simulink model "MX_Gateway"  (Simulink 9.0)
settings: Solver=FixedStepDiscrete  StartTime=0.0  StopTime=100  FixedStep=0.01  RelTol=1e-3
values from neighbouring files (symbols this model uses):
  ACTIVE = Bobcat.Parameter; CreateDefine( ACTIVE                         ,1                          ,'Define' ,'defines.h' )    [defines.m]
  ACTIVE_MODE_ONE = Bobcat.Parameter; CreateDefine( ACTIVE_MODE_ONE                ,19                         ,'Define' ,'defines.h' )    [defines.m]
  AUTHENTICATED = Bobcat.Parameter; CreateDefine( AUTHENTICATED                  ,1                          ,'Define' ,'defines.h' )    [defines.m]
  AUX_EMEA_CONTROLS = Bobcat.Parameter; CreateDefine( AUX_EMEA_CONTROLS  ,1   ,'Define' ,'defines.h' )    [enums.m]
  AUX_FLOW_HIGH = Bobcat.Parameter; CreateDefine( AUX_FLOW_HIGH           ,4  ,'Define' ,'defines.h' )    [enums.m]
  AUX_FLOW_INACTIVE = Bobcat.Parameter; CreateDefine( AUX_FLOW_INACTIVE       ,0  ,'Define' ,'defines.h' )    [enums.m]
  AUX_FLOW_LOW = Bobcat.Parameter; CreateDefine( AUX_FLOW_LOW            ,1  ,'Define' ,'defines.h' )    [enums.m]
  AUX_FLOW_MEDIUM = Bobcat.Parameter; CreateDefine( AUX_FLOW_MEDIUM         ,2  ,'Define' ,'defines.h' )    [enums.m]
  AUX_FLOW_STANDARD = Bobcat.Parameter; CreateDefine( AUX_FLOW_STANDARD       ,3  ,'Define' ,'defines.h' )    [enums.m]
  AUX_NON_FUNCTIONAL = Bobcat.Parameter; CreateDefine( AUX_NON_FUNCTIONAL ,255 ,'Define' ,'defines.h' )    [enums.m]
  BASE_ACTIVE = Bobcat.Parameter; CreateDefine( BASE_ACTIVE                    ,253                        ,'Define' ,'defines.h' )    [defines.m]
  BASE_DETENT = Bobcat.Parameter; CreateDefine( BASE_DETENT                    ,1                          ,'Define' ,'defines.h' )    [defines.m]
  BEEP_NONE = Bobcat.Parameter; CreateDefine( BEEP_NONE                      ,255                        ,'Define' ,'defines.h' )    [defines.m]
  CD_AIR_FILTER_DEBOUNCE_NORMAL = Bobcat.Parameter; CreateAliasCD( CD_AIR_FILTER_DEBOUNCE_NORMAL                        ,uint16(500)                ,'ConstantDataPointer' ,'CONSTANT_DATA' ,'AIR_FILTER_DEBOUNCE_NORMAL')    [cdata.m]    (also in hc12_cdata.m; m4_cdata.m)
  CD_AIR_FILTER_DEBOUNCE_NOT_CONNECTED = Bobcat.Parameter; CreateAliasCD( CD_AIR_FILTER_DEBOUNCE_NOT_CONNECTED                 ,uint16(3000)               ,'ConstantDataPointer' ,'CONSTANT_DATA' ,'AIR_FILTER_DEBOUNCE_NOT_CONNECTED')    [cdata.m]    (also in hc12_cdata.m; m4_cdata.m)
  CD_AIR_FILTER_DEBOUNCE_PLUGGED = Bobcat.Parameter; CreateAliasCD( CD_AIR_FILTER_DEBOUNCE_PLUGGED                       ,uint16(3000)               ,'ConstantDataPointer' ,'CONSTANT_DATA' ,'AIR_FILTER_DEBOUNCE_PLUGGED')    [cdata.m]    (also in hc12_cdata.m; m4_cdata.m)
  CD_AIR_FILTER_FUNCTION = Bobcat.Parameter; CreateAliasCD( CD_AIR_FILTER_FUNCTION                               ,uint8(255)                 ,'ConstantDataPointer' ,'CONSTANT_DATA' ,'AIR_FILTER_FUNCTION')    [cdata.m]    (also in hc12_cdata.m; m4_cdata.m)
  CD_AIR_FILTER_PLUGGED_WEIGHT = Bobcat.Parameter; CreateAliasCD( CD_AIR_FILTER_PLUGGED_WEIGHT                         ,uint8(2)                   ,'ConstantDataPointer' ,'CONSTANT_DATA' ,'AIR_FILTER_PLUGGED_WEIGHT')    [cdata.m]    (also in hc12_cdata.m; m4_cdata.m)
  CD_AIR_FILTER_UNPLUGGED_WEIGHT = Bobcat.Parameter; CreateAliasCD( CD_AIR_FILTER_UNPLUGGED_WEIGHT                       ,uint8(1)                   ,'ConstantDataPointer' ,'CONSTANT_DATA' ,'AIR_FILTER_UNPLUGGED_WEIGHT')    [cdata.m]    (also in hc12_cdata.m; m4_cdata.m)
  CD_AUTOIDLE_ARMBAR_DELAY = Bobcat.Parameter; CreateAliasCD( CD_AUTOIDLE_ARMBAR_DELAY                             ,uint8(2)                   ,'ConstantDataPointer' ,'CONSTANT_DATA' ,'AUTOIDLE_ARMBAR_DELAY')    [cdata.m]    (also in hc12_cdata.m; m4_cdata.m)
  CD_AUTOIDLE_FUNCTION = Bobcat.Parameter; CreateAliasCD( CD_AUTOIDLE_FUNCTION                                 ,uint8(255)                 ,'ConstantDataPointer' ,'CONSTANT_DATA' ,'AUTOIDLE_FUNCTION')    [cdata.m]    (also in hc12_cdata.m; m4_cdata.m)
  CD_AUTOIDLE_IDLE_DELAY = Bobcat.Parameter; CreateAliasCD( CD_AUTOIDLE_IDLE_DELAY                               ,uint8(40)                  ,'ConstantDataPointer' ,'CONSTANT_DATA' ,'AUTOIDLE_IDLE_DELAY')    [cdata.m]    (also in hc12_cdata.m; m4_cdata.m)
  CD_AUTOIDLE_LOW_IDLE_START = Bobcat.Parameter; CreateAliasCD( CD_AUTOIDLE_LOW_IDLE_START                           ,uint8(255)                 ,'ConstantDataPointer' ,'CONSTANT_DATA' ,'AUTOIDLE_LOW_IDLE_START')    [cdata.m]    (also in hc12_cdata.m; m4_cdata.m)
  CD_AUTOIDLE_PRESSURE_OFF = Bobcat.Parameter; CreateAliasCD( CD_AUTOIDLE_PRESSURE_OFF                             ,uint16(300)                ,'ConstantDataPointer' ,'CONSTANT_DATA' ,'AUTOIDLE_PRESSURE_OFF')    [cdata.m]    (also in hc12_cdata.m; m4_cdata.m)
  CD_AUTOIDLE_PRESSURE_ON = Bobcat.Parameter; CreateAliasCD( CD_AUTOIDLE_PRESSURE_ON                              ,uint16(270)                ,'ConstantDataPointer' ,'CONSTANT_DATA' ,'AUTOIDLE_PRESSURE_ON')    [cdata.m]    (also in hc12_cdata.m; m4_cdata.m)
  CD_AUTOSHIFT_FUNCTION = Bobcat.Parameter; CreateAliasCD( CD_AUTOSHIFT_FUNCTION                                ,uint8(255)                 ,'ConstantDataPointer' ,'CONSTANT_DATA' ,'AUTOSHIFT_FUNCTION')    [cdata.m]    (also in hc12_cdata.m; m4_cdata.m)
  CD_AUTOSHIFT_PHIGH1 = Bobcat.Parameter; CreateAliasCD( CD_AUTOSHIFT_PHIGH1                                  ,uint16(205)                ,'ConstantDataPointer' ,'CONSTANT_DATA' ,'AUTOSHIFT_PHIGH1')    [cdata.m]    (also in hc12_cdata.m; m4_cdata.m)
  CD_AUTOSHIFT_PHIGH2 = Bobcat.Parameter; CreateAliasCD( CD_AUTOSHIFT_PHIGH2                                  ,uint16(193)                ,'ConstantDataPointer' ,'CONSTANT_DATA' ,'AUTOSHIFT_PHIGH2')    [cdata.m]    (also in hc12_cdata.m; m4_cdata.m)
  CD_AUTOSHIFT_PHIGH_TEMP1 = Bobcat.Parameter; CreateAliasCD( CD_AUTOSHIFT_PHIGH_TEMP1                             ,int16(70)                  ,'ConstantDataPointer' ,'CONSTANT_DATA' ,'AUTOSHIFT_PHIGH_TEMP1')    [cdata.m]    (also in hc12_cdata.m; m4_cdata.m)
  CD_AUTOSHIFT_PHIGH_TEMP2 = Bobcat.Parameter; CreateAliasCD( CD_AUTOSHIFT_PHIGH_TEMP2                             ,int16(160)                 ,'ConstantDataPointer' ,'CONSTANT_DATA' ,'AUTOSHIFT_PHIGH_TEMP2')    [cdata.m]    (also in hc12_cdata.m; m4_cdata.m)
  CD_AUTOSHIFT_PLOW1 = Bobcat.Parameter; CreateAliasCD( CD_AUTOSHIFT_PLOW1                                   ,uint16(130)                ,'ConstantDataPointer' ,'CONSTANT_DATA' ,'AUTOSHIFT_PLOW1')    [cdata.m]    (also in hc12_cdata.m; m4_cdata.m)
  CD_AUTOSHIFT_PLOW2 = Bobcat.Parameter; CreateAliasCD( CD_AUTOSHIFT_PLOW2                                   ,uint16(100)                ,'ConstantDataPointer' ,'CONSTANT_DATA' ,'AUTOSHIFT_PLOW2')    [cdata.m]    (also in hc12_cdata.m; m4_cdata.m)
  CD_AUTOSHIFT_PLOW_TEMP1 = Bobcat.Parameter; CreateAliasCD( CD_AUTOSHIFT_PLOW_TEMP1                              ,int16(20)                  ,'ConstantDataPointer' ,'CONSTANT_DATA' ,'AUTOSHIFT_PLOW_TEMP1')    [cdata.m]    (also in hc12_cdata.m; m4_cdata.m)
  CD_AUTOSHIFT_PLOW_TEMP2 = Bobcat.Parameter; CreateAliasCD( CD_AUTOSHIFT_PLOW_TEMP2                              ,int16(80)                  ,'ConstantDataPointer' ,'CONSTANT_DATA' ,'AUTOSHIFT_PLOW_TEMP2')    [cdata.m]    (also in hc12_cdata.m; m4_cdata.m)
  CD_AUTOSHIFT_TRAVEL_DELAY = Bobcat.Parameter; CreateAliasCD( CD_AUTOSHIFT_TRAVEL_DELAY                            ,uint8(32)                  ,'ConstantDataPointer' ,'CONSTANT_DATA' ,'AUTOSHIFT_TRAVEL_DELAY')    [cdata.m]    (also in hc12_cdata.m; m4_cdata.m)
  CD_AUX2_CRACKPOINT_CURRENT = Bobcat.Parameter; CreateAliasCD( CD_AUX2_CRACKPOINT_CURRENT                           ,fi(0,0,16,12,'hex','0c28') ,'ConstantDataPointer' ,'CONSTANT_DATA' ,'AUX2_CRACKPOINT_CURRENT')    [cdata.m]    (also in hc12_cdata.m; m4_cdata.m)
  CD_AUX2_FLOW_LOW_FUNCTION = Bobcat.Parameter; CreateAliasCD( CD_AUX2_FLOW_LOW_FUNCTION                            ,uint8(255)                 ,'ConstantDataPointer' ,'CONSTANT_DATA' ,'AUX2_FLOW_LOW_FUNCTION')    [cdata.m]    (also in hc12_cdata.m; m4_cdata.m)
  CD_AUX2_FLOW_MED_FUNCTION = Bobcat.Parameter; CreateAliasCD( CD_AUX2_FLOW_MED_FUNCTION                            ,uint8(255)                 ,'ConstantDataPointer' ,'CONSTANT_DATA' ,'AUX2_FLOW_MED_FUNCTION')    [cdata.m]    (also in hc12_cdata.m; m4_cdata.m)
  CD_AUX2_HI_FLOW_PERCENT = Bobcat.Parameter; CreateAliasCD( CD_AUX2_HI_FLOW_PERCENT                              ,fi(0,0,16,16,'hex','ffff') ,'ConstantDataPointer' ,'CONSTANT_DATA' ,'AUX2_HI_FLOW_PERCENT')    [cdata.m]    (also in hc12_cdata.m; m4_cdata.m)
  CD_AUX2_LOW_FLOW_PERCENT = Bobcat.Parameter; CreateAliasCD( CD_AUX2_LOW_FLOW_PERCENT                             ,fi(0,0,16,16,'hex','7333') ,'ConstantDataPointer' ,'CONSTANT_DATA' ,'AUX2_LOW_FLOW_PERCENT')    [cdata.m]    (also in hc12_cdata.m; m4_cdata.m)
  CD_AUX2_MAX_FLOW_CURRENT = Bobcat.Parameter; CreateAliasCD( CD_AUX2_MAX_FLOW_CURRENT                             ,fi(0,0,16,12,'hex','14cc') ,'ConstantDataPointer' ,'CONSTANT_DATA' ,'AUX2_MAX_FLOW_CURRENT')    [cdata.m]    (also in hc12_cdata.m; m4_cdata.m)
  CD_AUX2_MED_FLOW_PERCENT = Bobcat.Parameter; CreateAliasCD( CD_AUX2_MED_FLOW_PERCENT                             ,fi(0,0,16,16,'hex','9999') ,'ConstantDataPointer' ,'CONSTANT_DATA' ,'AUX2_MED_FLOW_PERCENT')    [cdata.m]    (also in hc12_cdata.m; m4_cdata.m)
  CD_AUX_CRACKPOINT_CURRENT = Bobcat.Parameter; CreateAliasCD( CD_AUX_CRACKPOINT_CURRENT                            ,fi(0,0,16,12,'hex','0000') ,'ConstantDataPointer' ,'CONSTANT_DATA' ,'AUX_CRACKPOINT_CURRENT')    [cdata.m]    (also in hc12_cdata.m; m4_cdata.m)
  CD_AUX_DETENT_FLASH_TIME = Bobcat.Parameter; CreateAliasCD( CD_AUX_DETENT_FLASH_TIME                             ,uint16(72)                 ,'ConstantDataPointer' ,'CONSTANT_DATA' ,'AUX_DETENT_FLASH_TIME')    [cdata.m]    (also in hc12_cdata.m; m4_cdata.m)
  CD_AUX_DETENT_HOLD_TIME = Bobcat.Parameter; CreateAliasCD( CD_AUX_DETENT_HOLD_TIME                              ,uint16(100)                ,'ConstantDataPointer' ,'CONSTANT_DATA' ,'AUX_DETENT_HOLD_TIME')    [cdata.m]    (also in hc12_cdata.m; m4_cdata.m)
  CD_AUX_FULL_ON_AUX1 = Bobcat.Parameter; CreateAliasCD( CD_AUX_FULL_ON_AUX1                                  ,fi(0,0,16,12,'hex','10cd') ,'ConstantDataPointer' ,'CONSTANT_DATA' ,'AUX_FULL_ON_AUX1')    [cdata.m]    (also in hc12_cdata.m; m4_cdata.m)
  CD_AUX_FULL_ON_AUX2 = Bobcat.Parameter; CreateAliasCD( CD_AUX_FULL_ON_AUX2                                  ,fi(0,0,16,12,'hex','139d') ,'ConstantDataPointer' ,'CONSTANT_DATA' ,'AUX_FULL_ON_AUX2')    [cdata.m]    (also in hc12_cdata.m; m4_cdata.m)
  CD_AUX_FULL_ON_AUX3 = Bobcat.Parameter; CreateAliasCD( CD_AUX_FULL_ON_AUX3                                  ,fi(0,0,16,12,'hex','17d7') ,'ConstantDataPointer' ,'CONSTANT_DATA' ,'AUX_FULL_ON_AUX3')    [cdata.m]    (also in hc12_cdata.m; m4_cdata.m)
  CD_AUX_FUNCTION = Bobcat.Parameter; CreateAliasCD( CD_AUX_FUNCTION                                      ,uint8(0)                   ,'ConstantDataPointer' ,'CONSTANT_DATA' ,'AUX_FUNCTION')    [cdata.m]    (also in hc12_cdata.m; m4_cdata.m)
  CD_AUX_RATE_LIMIT_CRACKPOINT = Bobcat.Parameter; CreateAliasCD( CD_AUX_RATE_LIMIT_CRACKPOINT                         ,fi(0,0,16,12,'hex','0800') ,'ConstantDataPointer' ,'CONSTANT_DATA' ,'AUX_RATE_LIMIT_CRACKPOINT')    [cdata.m]    (also in hc12_cdata.m; m4_cdata.m)
  CD_AUX_RATE_LIMIT_CRACKPOINT_RATE = Bobcat.Parameter; CreateAliasCD( CD_AUX_RATE_LIMIT_CRACKPOINT_RATE                    ,fi(0,1,32,12,'int',102400) ,'ConstantDataPointer' ,'CONSTANT_DATA' ,'AUX_RATE_LIMIT_CRACKPOINT_RATE')    [cdata.m]    (also in hc12_cdata.m; m4_cdata.m)
  CD_AUX_RATE_LIMIT_FUNCTION = Bobcat.Parameter; CreateAliasCD( CD_AUX_RATE_LIMIT_FUNCTION                           ,uint8(255)                 ,'ConstantDataPointer' ,'CONSTANT_DATA' ,'AUX_RATE_LIMIT_FUNCTION')    [cdata.m]    (also in hc12_cdata.m; m4_cdata.m)
  CD_AUX_RATE_LIMIT_MAX_ENG_SPD = Bobcat.Parameter; CreateAliasCD( CD_AUX_RATE_LIMIT_MAX_ENG_SPD                        ,uint16(1600)               ,'ConstantDataPointer' ,'CONSTANT_DATA' ,'AUX_RATE_LIMIT_MAX_ENG_SPD')    [cdata.m]    (also in hc12_cdata.m; m4_cdata.m)
  CD_AUX_RATE_LIMIT_MIN_ENG_SPD = Bobcat.Parameter; CreateAliasCD( CD_AUX_RATE_LIMIT_MIN_ENG_SPD                        ,uint16(100)                ,'ConstantDataPointer' ,'CONSTANT_DATA' ,'AUX_RATE_LIMIT_MIN_ENG_SPD')    [cdata.m]    (also in hc12_cdata.m; m4_cdata.m)
  CD_AUX_RATE_LIMIT_NORMAL_RATE = Bobcat.Parameter; CreateAliasCD( CD_AUX_RATE_LIMIT_NORMAL_RATE                        ,fi(0,1,32,12,'int',2048)   ,'ConstantDataPointer' ,'CONSTANT_DATA' ,'AUX_RATE_LIMIT_NORMAL_RATE')    [cdata.m]    (also in hc12_cdata.m; m4_cdata.m)
  CD_AUXPOT_CAL_DEADBAND_HIGH = Bobcat.Parameter; CreateAliasCD( CD_AUXPOT_CAL_DEADBAND_HIGH                          ,uint16(574)                ,'ConstantDataPointer' ,'CONSTANT_DATA' ,'AUXPOT_CAL_DEADBAND_HIGH')    [cdata.m]    (also in hc12_cdata.m; m4_cdata.m)
  CD_AUXPOT_CAL_DEADBAND_LOW = Bobcat.Parameter; CreateAliasCD( CD_AUXPOT_CAL_DEADBAND_LOW                           ,uint16(424)                ,'ConstantDataPointer' ,'CONSTANT_DATA' ,'AUXPOT_CAL_DEADBAND_LOW')    [cdata.m]    (also in hc12_cdata.m; m4_cdata.m)
  CD_AUXPOT_CENTER_RATIO = Bobcat.Parameter; CreateAliasCD( CD_AUXPOT_CENTER_RATIO                               ,uint16(500)                ,'ConstantDataPointer' ,'CONSTANT_DATA' ,'AUXPOT_CENTER_RATIO')    [cdata.m]    (also in hc12_cdata.m; m4_cdata.m)
  CD_AUXPOT_DEADBAND = Bobcat.Parameter; CreateAliasCD( CD_AUXPOT_DEADBAND                                   ,uint8(38)                  ,'ConstantDataPointer' ,'CONSTANT_DATA' ,'AUXPOT_DEADBAND')    [cdata.m]    (also in hc12_cdata.m; m4_cdata.m)
  CD_AUXPOT_FUNCTION = Bobcat.Parameter; CreateAliasCD( CD_AUXPOT_FUNCTION                                   ,uint8(0)                   ,'ConstantDataPointer' ,'CONSTANT_DATA' ,'AUXPOT_FUNCTION')    [cdata.m]    (also in hc12_cdata.m; m4_cdata.m)
  CD_AUXPOT_RATIO_GAIN = Bobcat.Parameter; CreateAliasCD( CD_AUXPOT_RATIO_GAIN                                 ,uint16(1000)               ,'ConstantDataPointer' ,'CONSTANT_DATA' ,'AUXPOT_RATIO_GAIN')    [cdata.m]    (also in hc12_cdata.m; m4_cdata.m)
  CD_CAN_THROTTLE_FUNCTION = Bobcat.Parameter; CreateAliasCD( CD_CAN_THROTTLE_FUNCTION                             ,uint8(255)                 ,'ConstantDataPointer' ,'CONSTANT_DATA' ,'CAN_THROTTLE_FUNCTION')    [cdata.m]    (also in hc12_cdata.m; m4_cdata.m)
  CD_CAN_THROTTLE_REVERSE = Bobcat.Parameter; CreateAliasCD( CD_CAN_THROTTLE_REVERSE                              ,uint8(255)                 ,'ConstantDataPointer' ,'CONSTANT_DATA' ,'CAN_THROTTLE_REVERSE')    [cdata.m]    (also in hc12_cdata.m; m4_cdata.m)
  CD_CONSOLE_DEBOUNCE_ABSENT = Bobcat.Parameter; CreateAliasCD( CD_CONSOLE_DEBOUNCE_ABSENT                           ,uint16(12)                 ,'ConstantDataPointer' ,'CONSTANT_DATA' ,'CONSOLE_DEBOUNCE_ABSENT')    [cdata.m]    (also in hc12_cdata.m; m4_cdata.m)
  CD_CONSOLE_DEBOUNCE_OORH = Bobcat.Parameter; CreateAliasCD( CD_CONSOLE_DEBOUNCE_OORH                             ,uint16(12)                 ,'ConstantDataPointer' ,'CONSTANT_DATA' ,'CONSOLE_DEBOUNCE_OORH')    [cdata.m]    (also in hc12_cdata.m; m4_cdata.m)
  CD_CONSOLE_DEBOUNCE_OORL = Bobcat.Parameter; CreateAliasCD( CD_CONSOLE_DEBOUNCE_OORL                             ,uint16(12)                 ,'ConstantDataPointer' ,'CONSTANT_DATA' ,'CONSOLE_DEBOUNCE_OORL')    [cdata.m]    (also in hc12_cdata.m; m4_cdata.m)
  CD_CONSOLE_DEBOUNCE_PRESENT = Bobcat.Parameter; CreateAliasCD( CD_CONSOLE_DEBOUNCE_PRESENT                          ,uint16(12)                 ,'ConstantDataPointer' ,'CONSTANT_DATA' ,'CONSOLE_DEBOUNCE_PRESENT')    [cdata.m]    (also in hc12_cdata.m; m4_cdata.m)
  CD_CONSOLE_FUNCTION = Bobcat.Parameter; CreateAliasCD( CD_CONSOLE_FUNCTION                                  ,uint8(0)                   ,'ConstantDataPointer' ,'CONSTANT_DATA' ,'CONSOLE_FUNCTION')    [cdata.m]    (also in hc12_cdata.m; m4_cdata.m)
  CD_CONSOLE_OORH = Bobcat.Parameter; CreateAliasCD( CD_CONSOLE_OORH                                      ,uint16(740)                ,'ConstantDataPointer' ,'CONSTANT_DATA' ,'CONSOLE_OORH')    [cdata.m]    (also in hc12_cdata.m; m4_cdata.m)
  CD_CONSOLE_OORL = Bobcat.Parameter; CreateAliasCD( CD_CONSOLE_OORL                                      ,uint16(10)                 ,'ConstantDataPointer' ,'CONSTANT_DATA' ,'CONSOLE_OORL')    [cdata.m]    (also in hc12_cdata.m; m4_cdata.m)
  CD_CONSOLE_PRESENT_HIGH = Bobcat.Parameter; CreateAliasCD( CD_CONSOLE_PRESENT_HIGH                              ,uint16(515)                ,'ConstantDataPointer' ,'CONSTANT_DATA' ,'CONSOLE_PRESENT_HIGH')    [cdata.m]    (also in hc12_cdata.m; m4_cdata.m)
  CD_CONSOLE_PRESENT_LOW = Bobcat.Parameter; CreateAliasCD( CD_CONSOLE_PRESENT_LOW                               ,uint16(375)                ,'ConstantDataPointer' ,'CONSTANT_DATA' ,'CONSOLE_PRESENT_LOW')    [cdata.m]    (also in hc12_cdata.m; m4_cdata.m)
  CD_DELUXE_GEN5_5_FUNCTION = Bobcat.Parameter; CreateAliasCD( CD_DELUXE_GEN5_5_FUNCTION                            ,uint8(255)                 ,'ConstantDataPointer' ,'CONSTANT_DATA' ,'DELUXE_GEN5_5_FUNCTION')    [cdata.m]    (also in hc12_cdata.m; m4_cdata.m)
  CD_DELUXE_GEN5_FUNCTION = Bobcat.Parameter; CreateAliasCD( CD_DELUXE_GEN5_FUNCTION                              ,uint8(255)                 ,'ConstantDataPointer' ,'CONSTANT_DATA' ,'DELUXE_GEN5_FUNCTION')    [cdata.m]    (also in hc12_cdata.m; m4_cdata.m)
  CD_DERATE_ENGINE_SPEED_DEBOUNCE_HIGH = Bobcat.Parameter; CreateAliasCD( CD_DERATE_ENGINE_SPEED_DEBOUNCE_HIGH                 ,uint16(200)                ,'ConstantDataPointer' ,'CONSTANT_DATA' ,'DERATE_ENGINE_SPEED_DEBOUNCE_HIGH')    [cdata.m]    (also in hc12_cdata.m; m4_cdata.m)
  CD_DERATE_ENGINE_SPEED_DEBOUNCE_SHUTDOWN = Bobcat.Parameter; CreateAliasCD( CD_DERATE_ENGINE_SPEED_DEBOUNCE_SHUTDOWN             ,uint16(1000)               ,'ConstantDataPointer' ,'CONSTANT_DATA' ,'DERATE_ENGINE_SPEED_DEBOUNCE_SHUTDOWN')    [cdata.m]    (also in hc12_cdata.m; m4_cdata.m)
  CD_DERATE_ENGINE_SPEED_HIGH_OFFSET = Bobcat.Parameter; CreateAliasCD( CD_DERATE_ENGINE_SPEED_HIGH_OFFSET                   ,uint16(200)                ,'ConstantDataPointer' ,'CONSTANT_DATA' ,'DERATE_ENGINE_SPEED_HIGH_OFFSET')    [cdata.m]    (also in hc12_cdata.m; m4_cdata.m)
  CD_DERATE_METHOD = Bobcat.Parameter; CreateAliasCD( CD_DERATE_METHOD                                     ,uint8(0)                   ,'ConstantDataPointer' ,'CONSTANT_DATA' ,'DERATE_METHOD')    [cdata.m]    (also in hc12_cdata.m; m4_cdata.m)
  CD_DUMP_VALVE_FUNCTION = Bobcat.Parameter; CreateAliasCD( CD_DUMP_VALVE_FUNCTION                               ,uint8(255)                 ,'ConstantDataPointer' ,'CONSTANT_DATA' ,'DUMP_VALVE_FUNCTION')    [cdata.m]    (also in hc12_cdata.m; m4_cdata.m)
  CD_DUMP_VALVE_ON_CURRENT = Bobcat.Parameter; CreateAliasCD( CD_DUMP_VALVE_ON_CURRENT                             ,fi(0,0,16,12,'hex','ffff') ,'ConstantDataPointer' ,'CONSTANT_DATA' ,'DUMP_VALVE_ON_CURRENT')    [cdata.m]    (also in hc12_cdata.m; m4_cdata.m)
  CD_ECO_MODE_THROTTLE_REDUCTION = Bobcat.Parameter; CreateAliasCD( CD_ECO_MODE_THROTTLE_REDUCTION                       ,uint16(850)                ,'ConstantDataPointer' ,'CONSTANT_DATA' ,'ECO_MODE_THROTTLE_REDUCTION')    [cdata.m]    (also in hc12_cdata.m; m4_cdata.m)
  CD_ECT_FILTER_GAIN = Bobcat.Parameter; CreateAliasCD( CD_ECT_FILTER_GAIN                                   ,uint8(6)                   ,'ConstantDataPointer' ,'CONSTANT_DATA' ,'ECT_FILTER_GAIN')    [cdata.m]    (also in hc12_cdata.m; m4_cdata.m)
  CD_ECU_FUNCTION = Bobcat.Parameter; CreateAliasCD( CD_ECU_FUNCTION                                      ,uint8(255)                 ,'ConstantDataPointer' ,'CONSTANT_DATA' ,'ECU_FUNCTION')    [cdata.m]    (also in hc12_cdata.m; m4_cdata.m)
  CD_ECU_HOLD_DEBOUNCE_ERROR_ON = Bobcat.Parameter; CreateAliasCD( CD_ECU_HOLD_DEBOUNCE_ERROR_ON                        ,uint16(20)                 ,'ConstantDataPointer' ,'CONSTANT_DATA' ,'ECU_HOLD_DEBOUNCE_ERROR_ON')    [cdata.m]    (also in hc12_cdata.m; m4_cdata.m)
  CD_EMEA_CONTROLS_HOLD_TIME = Bobcat.Parameter; CreateAliasCD( CD_EMEA_CONTROLS_HOLD_TIME                           ,uint16(30)                 ,'ConstantDataPointer' ,'CONSTANT_DATA' ,'EMEA_CONTROLS_HOLD_TIME')    [cdata.m]    (also in hc12_cdata.m; m4_cdata.m)
  CD_EMEA_CONTROLS_OPTION_A = Bobcat.Parameter; CreateAliasCD( CD_EMEA_CONTROLS_OPTION_A                            ,uint8(255)                 ,'ConstantDataPointer' ,'CONSTANT_DATA' ,'EMEA_CONTROLS_OPTION_A')    [cdata.m]    (also in hc12_cdata.m; m4_cdata.m)
  CD_EMEA_CONTROLS_OPTION_C = Bobcat.Parameter; CreateAliasCD( CD_EMEA_CONTROLS_OPTION_C                            ,uint8(255)                 ,'ConstantDataPointer' ,'CONSTANT_DATA' ,'EMEA_CONTROLS_OPTION_C')    [cdata.m]    (also in hc12_cdata.m; m4_cdata.m)
  CD_EMEA_CONTROLS_REVERSE = Bobcat.Parameter; CreateAliasCD( CD_EMEA_CONTROLS_REVERSE                             ,uint8(255)                 ,'ConstantDataPointer' ,'CONSTANT_DATA' ,'EMEA_CONTROLS_REVERSE')    [cdata.m]    (also in hc12_cdata.m; m4_cdata.m)
  CD_EMEA_ELECTRIC_VERSION = Bobcat.Parameter; CreateAliasCD( CD_EMEA_ELECTRIC_VERSION                             ,uint8(0)                   ,'ConstantDataPointer' ,'CONSTANT_DATA' ,'EMEA_ELECTRIC_VERSION')    [cdata.m]    (also in hc12_cdata.m; m4_cdata.m)
  CD_EMEA_POT_CAL_DEADBAND_HIGH = Bobcat.Parameter; CreateAliasCD( CD_EMEA_POT_CAL_DEADBAND_HIGH                        ,uint16(574)                ,'ConstantDataPointer' ,'CONSTANT_DATA' ,'EMEA_POT_CAL_DEADBAND_HIGH')    [cdata.m]    (also in hc12_cdata.m; m4_cdata.m)
  CD_EMEA_POT_CAL_DEADBAND_LOW = Bobcat.Parameter; CreateAliasCD( CD_EMEA_POT_CAL_DEADBAND_LOW                         ,uint16(424)                ,'ConstantDataPointer' ,'CONSTANT_DATA' ,'EMEA_POT_CAL_DEADBAND_LOW')    [cdata.m]    (also in hc12_cdata.m; m4_cdata.m)
  CD_ENGINE_RPM_FACTOR = Bobcat.Parameter; CreateAliasCD( CD_ENGINE_RPM_FACTOR                                 ,uint8(35)                  ,'ConstantDataPointer' ,'CONSTANT_DATA' ,'ENGINE_RPM_FACTOR')    [cdata.m]    (also in hc12_cdata.m; m4_cdata.m)
  CD_ENGINE_SPEED_FILTER_FAST_VALUE = Bobcat.Parameter; CreateAliasCD( CD_ENGINE_SPEED_FILTER_FAST_VALUE                    ,uint8(1)                   ,'ConstantDataPointer' ,'CONSTANT_DATA' ,'ENGINE_SPEED_FILTER_FAST_VALUE')    [cdata.m]    (also in hc12_cdata.m; m4_cdata.m)
  CD_ENGINE_SPEED_FILTER_SLOW_VALUE = Bobcat.Parameter; CreateAliasCD( CD_ENGINE_SPEED_FILTER_SLOW_VALUE                    ,uint8(4)                   ,'ConstantDataPointer' ,'CONSTANT_DATA' ,'ENGINE_SPEED_FILTER_SLOW_VALUE')    [cdata.m]    (also in hc12_cdata.m; m4_cdata.m)
  CD_ENGINE_SPEED_RUNNING = Bobcat.Parameter; CreateAliasCD( CD_ENGINE_SPEED_RUNNING                              ,uint16(800)                ,'ConstantDataPointer' ,'CONSTANT_DATA' ,'ENGINE_SPEED_RUNNING')    [cdata.m]    (also in hc12_cdata.m; m4_cdata.m)
  CD_ENGINE_START_DELAY_DEBOUNCE_RPM_NO_SIGNAL = Bobcat.Parameter; CreateAliasCD( CD_ENGINE_START_DELAY_DEBOUNCE_RPM_NO_SIGNAL         ,uint16(0)                  ,'ConstantDataPointer' ,'CONSTANT_DATA' ,'ENGINE_START_DELAY_DEBOUNCE_RPM_NO_SIGNAL')    [cdata.m]    (also in hc12_cdata.m; m4_cdata.m)
  CD_ENGINE_START_DELAY_OFF_TIME = Bobcat.Parameter; CreateAliasCD( CD_ENGINE_START_DELAY_OFF_TIME                       ,uint16(0)                  ,'ConstantDataPointer' ,'CONSTANT_DATA' ,'ENGINE_START_DELAY_OFF_TIME')    [cdata.m]    (also in hc12_cdata.m; m4_cdata.m)
  CD_ENGINE_START_DELAY_RPM_NO_SIGNAL = Bobcat.Parameter; CreateAliasCD( CD_ENGINE_START_DELAY_RPM_NO_SIGNAL                  ,uint16(0)                  ,'ConstantDataPointer' ,'CONSTANT_DATA' ,'ENGINE_START_DELAY_RPM_NO_SIGNAL')    [cdata.m]    (also in hc12_cdata.m; m4_cdata.m)
  CD_EOP_DEBOUNCE_EXTREMELY_LOW = Bobcat.Parameter; CreateAliasCD( CD_EOP_DEBOUNCE_EXTREMELY_LOW                        ,uint16(50)                 ,'ConstantDataPointer' ,'CONSTANT_DATA' ,'EOP_DEBOUNCE_EXTREMELY_LOW')    [cdata.m]    (also in hc12_cdata.m; m4_cdata.m)
  CD_EOP_DEBOUNCE_NORMAL = Bobcat.Parameter; CreateAliasCD( CD_EOP_DEBOUNCE_NORMAL                               ,uint16(50)                 ,'ConstantDataPointer' ,'CONSTANT_DATA' ,'EOP_DEBOUNCE_NORMAL')    [cdata.m]    (also in hc12_cdata.m; m4_cdata.m)
  CD_EOP_DEBOUNCE_SHUTDOWN = Bobcat.Parameter; CreateAliasCD( CD_EOP_DEBOUNCE_SHUTDOWN                             ,uint16(100)                ,'ConstantDataPointer' ,'CONSTANT_DATA' ,'EOP_DEBOUNCE_SHUTDOWN')    [cdata.m]    (also in hc12_cdata.m; m4_cdata.m)
  CD_EOP_FILTER_VALUE = Bobcat.Parameter; CreateAliasCD( CD_EOP_FILTER_VALUE                                  ,uint8(3)                   ,'ConstantDataPointer' ,'CONSTANT_DATA' ,'EOP_FILTER_VALUE')    [cdata.m]    (also in hc12_cdata.m; m4_cdata.m)
  CD_EOP_FUNCTION = Bobcat.Parameter; CreateAliasCD( CD_EOP_FUNCTION                                      ,uint8(1)                   ,'ConstantDataPointer' ,'CONSTANT_DATA' ,'EOP_FUNCTION')    [cdata.m]    (also in hc12_cdata.m; m4_cdata.m)
  CD_EOP_SWITCH_GAUGE_VALUE = Bobcat.Parameter; CreateAliasCD( CD_EOP_SWITCH_GAUGE_VALUE                            ,uint16(125)                ,'ConstantDataPointer' ,'CONSTANT_DATA' ,'EOP_SWITCH_GAUGE_VALUE')    [cdata.m]    (also in hc12_cdata.m; m4_cdata.m)
  CD_EOP_SWITCH_LOW = Bobcat.Parameter; CreateAliasCD( CD_EOP_SWITCH_LOW                                    ,uint16(50)                 ,'ConstantDataPointer' ,'CONSTANT_DATA' ,'EOP_SWITCH_LOW')    [cdata.m]    (also in hc12_cdata.m; m4_cdata.m)
  CD_EOP_SWITCH_PRESSURE_VALUE = Bobcat.Parameter; CreateAliasCD( CD_EOP_SWITCH_PRESSURE_VALUE                         ,uint16(40)                 ,'ConstantDataPointer' ,'CONSTANT_DATA' ,'EOP_SWITCH_PRESSURE_VALUE')    [cdata.m]    (also in hc12_cdata.m; m4_cdata.m)
  CD_EOT_DEBOUNCE_EXTREMELY_LOW = Bobcat.Parameter; CreateAliasCD( CD_EOT_DEBOUNCE_EXTREMELY_LOW                        ,uint16(2)                  ,'ConstantDataPointer' ,'CONSTANT_DATA' ,'EOT_DEBOUNCE_EXTREMELY_LOW')    [cdata.m]    (also in hc12_cdata.m; m4_cdata.m)
  CD_EOT_DEBOUNCE_LOW = Bobcat.Parameter; CreateAliasCD( CD_EOT_DEBOUNCE_LOW                                  ,uint16(2)                  ,'ConstantDataPointer' ,'CONSTANT_DATA' ,'EOT_DEBOUNCE_LOW')    [cdata.m]    (also in hc12_cdata.m; m4_cdata.m)
  CD_EOT_DEBOUNCE_NORMAL = Bobcat.Parameter; CreateAliasCD( CD_EOT_DEBOUNCE_NORMAL                               ,uint16(2)                  ,'ConstantDataPointer' ,'CONSTANT_DATA' ,'EOT_DEBOUNCE_NORMAL')    [cdata.m]    (also in hc12_cdata.m; m4_cdata.m)
  CD_EOT_EXTREMELY_LOW = Bobcat.Parameter; CreateAliasCD( CD_EOT_EXTREMELY_LOW                                 ,uint16(81)                 ,'ConstantDataPointer' ,'CONSTANT_DATA' ,'EOT_EXTREMELY_LOW')    [cdata.m]    (also in hc12_cdata.m; m4_cdata.m)
  CD_EOT_FUNCTION = Bobcat.Parameter; CreateAliasCD( CD_EOT_FUNCTION                                      ,uint8(255)                 ,'ConstantDataPointer' ,'CONSTANT_DATA' ,'EOT_FUNCTION')    [cdata.m]    (also in hc12_cdata.m; m4_cdata.m)
  CD_EOT_LOW = Bobcat.Parameter; CreateAliasCD( CD_EOT_LOW                                           ,uint16(90)                 ,'ConstantDataPointer' ,'CONSTANT_DATA' ,'EOT_LOW')    [cdata.m]    (also in hc12_cdata.m; m4_cdata.m)
  CD_EOT_TEMPERATURE_HYSTERESIS = Bobcat.Parameter; CreateAliasCD( CD_EOT_TEMPERATURE_HYSTERESIS                        ,uint16(2)                  ,'ConstantDataPointer' ,'CONSTANT_DATA' ,'EOT_TEMPERATURE_HYSTERESIS')    [cdata.m]    (also in hc12_cdata.m; m4_cdata.m)
  CD_FAST_TRAVEL_FUNCTION = Bobcat.Parameter; CreateAliasCD( CD_FAST_TRAVEL_FUNCTION                              ,uint8(255)                 ,'ConstantDataPointer' ,'CONSTANT_DATA' ,'FAST_TRAVEL_FUNCTION')    [cdata.m]    (also in hc12_cdata.m; m4_cdata.m)
  CD_FAST_TRAVEL_THROTTLE_DELAY = Bobcat.Parameter; CreateAliasCD( CD_FAST_TRAVEL_THROTTLE_DELAY                        ,uint16(10)                 ,'ConstantDataPointer' ,'CONSTANT_DATA' ,'FAST_TRAVEL_THROTTLE_DELAY')    [cdata.m]    (also in hc12_cdata.m; m4_cdata.m)
  CD_FAST_TRAVEL_THROTTLE_LIMIT = Bobcat.Parameter; CreateAliasCD( CD_FAST_TRAVEL_THROTTLE_LIMIT                        ,uint16(1000)               ,'ConstantDataPointer' ,'CONSTANT_DATA' ,'FAST_TRAVEL_THROTTLE_LIMIT')    [cdata.m]    (also in hc12_cdata.m; m4_cdata.m)
  CD_FLYWHEEL_TEETH = Bobcat.Parameter; CreateAliasCD( CD_FLYWHEEL_TEETH                                    ,uint8(83)                  ,'ConstantDataPointer' ,'CONSTANT_DATA' ,'FLYWHEEL_TEETH')    [cdata.m]    (also in hc12_cdata.m; m4_cdata.m)
  CD_FUEL_LEVEL_FILTER_FAST_VALUE = Bobcat.Parameter; CreateAliasCD( CD_FUEL_LEVEL_FILTER_FAST_VALUE                      ,uint8(5)                   ,'ConstantDataPointer' ,'CONSTANT_DATA' ,'FUEL_LEVEL_FILTER_FAST_VALUE')    [cdata.m]    (also in hc12_cdata.m; m4_cdata.m)
  CD_FUEL_LEVEL_FILTER_SLOW_VALUE = Bobcat.Parameter; CreateAliasCD( CD_FUEL_LEVEL_FILTER_SLOW_VALUE                      ,uint8(10)                  ,'ConstantDataPointer' ,'CONSTANT_DATA' ,'FUEL_LEVEL_FILTER_SLOW_VALUE')    [cdata.m]    (also in hc12_cdata.m; m4_cdata.m)
  CD_FUEL_PRIMARY_FEEDBACK_FUNCTION = Bobcat.Parameter; CreateAliasCD( CD_FUEL_PRIMARY_FEEDBACK_FUNCTION                    ,uint8(0)                   ,'ConstantDataPointer' ,'CONSTANT_DATA' ,'FUEL_PRIMARY_FEEDBACK_FUNCTION')    [cdata.m]    (also in hc12_cdata.m; m4_cdata.m)
  ... (+246 more)
undefined symbols (not defined in the file or in its folder): sigbuilder_block
undefined symbols (not defined in the file): CD_FUEL_RESOLUTION_TIMER
libraries: simulink x186, MX_Library x107
(part 21 of 48)

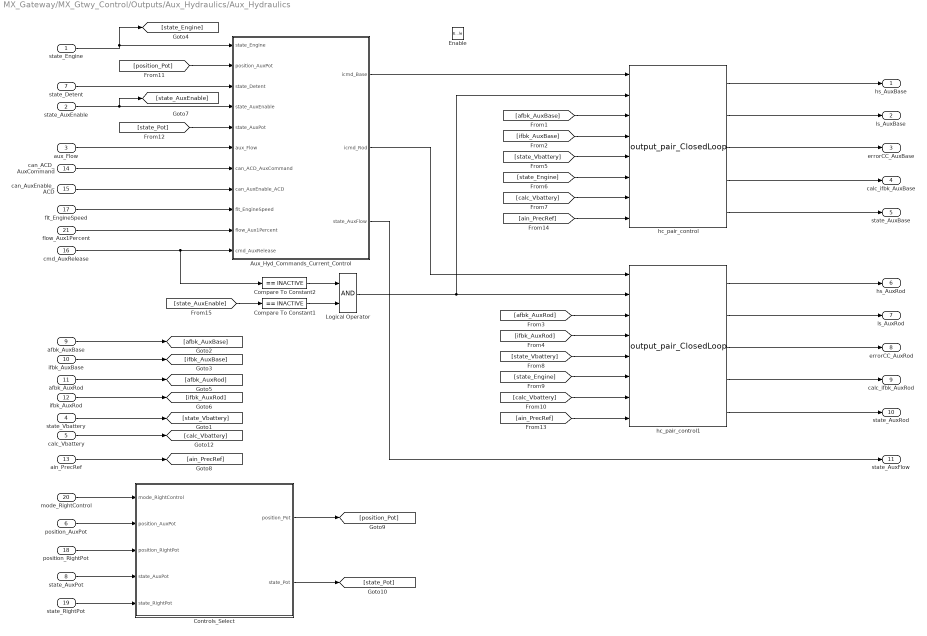
== system MX_Gateway/MX_Gtwy_Control/Outputs/Aux_Hydraulics/Aux_Hydraulics ==
  state_Engine : Inport
  state_AuxEnable : Inport  Port=2
  aux_Flow : Inport  Port=3
  state_Vbattery : Inport  Port=4
  calc_Vbattery : Inport  Port=5
  position_AuxPot : Inport  Port=6
  state_Detent : Inport  Port=7
  state_AuxPot : Inport  Port=8
  afbk_AuxBase : Inport  Port=9
  ifbk_AuxBase : Inport  Port=10
  afbk_AuxRod : Inport  Port=11
  ifbk_AuxRod : Inport  Port=12
  ain_PrecRef : Inport  Port=13
  can_ACD_AuxCommand : Inport  Port=14
  can_AuxEnable_ACD : Inport  Port=15
  cmd_AuxRelease : Inport  Port=16
  flt_EngineSpeed : Inport  Port=17
  position_RightPot : Inport  Port=18
  state_RightPot : Inport  Port=19
  mode_RightControl : Inport  Port=20
  flow_Aux1Percent : Inport  Port=21
  Enable : EnablePort  StatesWhenEnabling=reset
  Aux_Hyd_Commands_Current_Control : Subsystem
  Compare To Constant1 : simulink/Logic and Bit Operations/Compare To Constant  relop===  const=INACTIVE  OutDataTypeStr=boolean
  Compare To Constant2 : simulink/Logic and Bit Operations/Compare To Constant  relop===  const=INACTIVE  OutDataTypeStr=boolean
  Controls_Select : Truth Table  TreatAsAtomicUnit=on
  Logical Operator : Logic  AllPortsSameDT=off  OutDataTypeStr=boolean
  hc_pair_control : MX_Library/output_pair_ClosedLoop  AFBK_GAIN=CD_AUX_AFBK_GAIN  IFBK_GAIN=CD_AUX_IFBK_GAIN  OPEN_DB_CNT=CD_AUX_DEBOUNCE_OPEN_CIRCUIT  STG_DB_CNT=CD_AUX_DEBOUNCE_SHORT_TO_GROUND  OSTG_DB_CNT=CD_AUX_DEBOUNCE_SHORT_TO_GROUND  STB_DB_CNT=CD_AUX_DEBOUNCE_SHORT_TO_BATTERY  OC_DB_CNT=CD_AUX_DEBOUNCE_OVERCURRENT  IFBK_MAX=CD_AUX_OVERCURRENT  NZ_IFBK=CD_AUX_NON_ZERO_CURRENT  NZ_DUTY=CD_AUX_NON_ZERO_DUTY  KP=CD_AUX_KP  KI=CD_AUX_KI  BIDIRECTIONAL_CTRL=NON_FUNCTIONAL
  hc_pair_control1 : MX_Library/output_pair_ClosedLoop  AFBK_GAIN=CD_AUX_AFBK_GAIN  IFBK_GAIN=CD_AUX_IFBK_GAIN  OPEN_DB_CNT=CD_AUX_DEBOUNCE_OPEN_CIRCUIT  STG_DB_CNT=CD_AUX_DEBOUNCE_SHORT_TO_GROUND  OSTG_DB_CNT=CD_AUX_DEBOUNCE_SHORT_TO_GROUND  STB_DB_CNT=CD_AUX_DEBOUNCE_SHORT_TO_BATTERY  OC_DB_CNT=CD_AUX_DEBOUNCE_OVERCURRENT  IFBK_MAX=CD_AUX_OVERCURRENT  NZ_IFBK=CD_AUX_NON_ZERO_CURRENT  NZ_DUTY=CD_AUX_NON_ZERO_DUTY  KP=CD_AUX_KP  KI=CD_AUX_KI  BIDIRECTIONAL_CTRL=NON_FUNCTIONAL
  hs_AuxBase : Outport  OutputWhenDisabled=reset  InitialOutput=[0]
  ls_AuxBase : Outport  Port=2  OutputWhenDisabled=reset  InitialOutput=[0]
  errorCC_AuxBase : Outport  Port=3  OutputWhenDisabled=reset  InitialOutput=[0]
  calc_ifbk_AuxBase : Outport  Port=4  OutputWhenDisabled=reset  InitialOutput=[0]
  state_AuxBase : Outport  Port=5  OutputWhenDisabled=reset  InitialOutput=[0]
  hs_AuxRod : Outport  Port=6  OutputWhenDisabled=reset  InitialOutput=[0]
  ls_AuxRod : Outport  Port=7  OutputWhenDisabled=reset  InitialOutput=[0]
  errorCC_AuxRod : Outport  Port=8  OutputWhenDisabled=reset  InitialOutput=[0]
  calc_ifbk_AuxRod : Outport  Port=9  OutputWhenDisabled=reset  InitialOutput=[0]
  state_AuxRod : Outport  Port=10  InitialOutput=[0]
  state_AuxFlow : Outport  Port=11  OutputWhenDisabled=reset  InitialOutput=[0]
  wires:
  state_Engine/1 -> [state_Engine], Aux_Hyd_Commands_Current_Control/1(state_Engine)
  state_Detent/1 -> Aux_Hyd_Commands_Current_Control/3(state_Detent)
  state_AuxEnable/1 -> Aux_Hyd_Commands_Current_Control/4(state_AuxEnable), [state_AuxEnable]
  aux_Flow/1 -> Aux_Hyd_Commands_Current_Control/6(aux_Flow)
  Aux_Hyd_Commands_Current_Control/1(icmd_Base) -> hc_pair_control/1
  afbk_AuxBase/1 -> [afbk_AuxBase]
  ifbk_AuxBase/1 -> [ifbk_AuxBase]
  state_Vbattery/1 -> [state_Vbattery]
  calc_Vbattery/1 -> [calc_Vbattery]
  afbk_AuxRod/1 -> [afbk_AuxRod]
  ifbk_AuxRod/1 -> [ifbk_AuxRod]
  [afbk_AuxBase] -> hc_pair_control/3
  [ifbk_AuxBase] -> hc_pair_control/4
  [state_Vbattery] -> hc_pair_control/5
  [state_Engine] -> hc_pair_control/6
  [calc_Vbattery] -> hc_pair_control/7
  hc_pair_control/1 -> hs_AuxBase/1
  hc_pair_control/2 -> ls_AuxBase/1
  hc_pair_control/3 -> errorCC_AuxBase/1
  hc_pair_control/4 -> calc_ifbk_AuxBase/1
  hc_pair_control/5 -> state_AuxBase/1
  [afbk_AuxRod] -> hc_pair_control1/3
  [ifbk_AuxRod] -> hc_pair_control1/4
  [state_Vbattery] -> hc_pair_control1/5
  [state_Engine] -> hc_pair_control1/6
  [calc_Vbattery] -> hc_pair_control1/7
  hc_pair_control1/1 -> hs_AuxRod/1
  hc_pair_control1/2 -> ls_AuxRod/1
  hc_pair_control1/3 -> errorCC_AuxRod/1
  hc_pair_control1/4 -> calc_ifbk_AuxRod/1
  hc_pair_control1/5 -> state_AuxRod/1
  ain_PrecRef/1 -> [ain_PrecRef]
  [ain_PrecRef] -> hc_pair_control1/8
  [ain_PrecRef] -> hc_pair_control/8
  can_ACD_AuxCommand/1 -> Aux_Hyd_Commands_Current_Control/7(can_ACD_AuxCommand)
  can_AuxEnable_ACD/1 -> Aux_Hyd_Commands_Current_Control/8(can_AuxEnable_ACD)
  cmd_AuxRelease/1 -> Aux_Hyd_Commands_Current_Control/11(cmd_AuxRelease), Compare To Constant2/1
  [state_AuxEnable] -> Compare To Constant1/1
  Compare To Constant1/1 -> Logical Operator/2
  Compare To Constant2/1 -> Logical Operator/1
  Logical Operator/1 -> hc_pair_control1/2, hc_pair_control/2
  Aux_Hyd_Commands_Current_Control/2(icmd_Rod) -> hc_pair_control1/1
  Aux_Hyd_Commands_Current_Control/3(state_AuxFlow) -> state_AuxFlow/1
  flt_EngineSpeed/1 -> Aux_Hyd_Commands_Current_Control/9(flt_EngineSpeed)
  [state_Pot] -> Aux_Hyd_Commands_Current_Control/5(state_AuxPot)
  [position_Pot] -> Aux_Hyd_Commands_Current_Control/2(position_AuxPot)
  state_RightPot/1 -> Controls_Select/5(state_RightPot)
  state_AuxPot/1 -> Controls_Select/4(state_AuxPot)
  position_RightPot/1 -> Controls_Select/3(position_RightPot)
  position_AuxPot/1 -> Controls_Select/2(position_AuxPot)
  mode_RightControl/1 -> Controls_Select/1(mode_RightControl)
  Controls_Select/1(position_Pot) -> [position_Pot]
  Controls_Select/2(state_Pot) -> [state_Pot]
  flow_Aux1Percent/1 -> Aux_Hyd_Commands_Current_Control/10(flow_Aux1Percent)

== system MX_Gateway/MX_Gtwy_Control/Outputs/Diverter/Diverter ==
  state_Engine : Inport
  state_Console : Inport  Port=2
  state_Vbattery : Inport  Port=3
  afbk_Diverter : Inport  Port=4
  ifbk_Diverter : Inport  Port=5
  calc_Vbattery : Inport  Port=6
  ain_PrecRef : Inport  Port=7
  din_SecondAuxSw : Inport  Port=8
  mode_LeftControl : Inport  Port=9
  Enable : EnablePort  StatesWhenEnabling=reset  PropagateVarSize=During execution
  Compare To Constant : simulink/Logic and Bit Operations/Compare To Constant  relop=~=  const=ACTIVE  OutDataTypeStr=boolean
  Diverter : Stateflow chart  TreatAsAtomicUnit=on
  Unit Delay : UnitDelay  SampleTime=-1
  output_pair_open_loop : MX_Library/output_pair_OpenLoop  FEEDBACK_ENABLE=CD_SECOND_AUX_FEEDBACK_FUNCTION  AFBK_GAIN=CD_SECOND_AUX_AFBK_GAIN  IFBK_GAIN=CD_SECOND_AUX_IFBK_GAIN  OPEN_DB_CNT=CD_SECOND_AUX_DEBOUNCE_OPEN_CIRCUIT  STG_DB_CNT=CD_SECOND_AUX_DEBOUNCE_SHORT_TO_GROUND  STB_DB_CNT=CD_SECOND_AUX_DEBOUNCE_SHORT_TO_BATTERY  OC_DB_CNT=CD_SECOND_AUX_DEBOUNCE_OVERCURRENT  OSTG_DB_CNT=CD_SECOND_AUX_DEBOUNCE_SHORT_TO_GROUND  IFBK_MAX=CD_SECOND_AUX_OVERCURRENT  NZ_IFBK=CD_SECOND_AUX_NON_ZERO_CURRENT  NZ_DUTY=CD_SECOND_AUX_NON_ZERO_DUTY  MAX_VOLTS=CD_SECOND_AUX_MAX_VOLTS
  state_Diverter : Outport  OutputWhenDisabled=reset  InitialOutput=[0]
  hs_Diverter : Outport  Port=2  OutputWhenDisabled=reset  InitialOutput=[0]
  ls_Diverter : Outport  Port=3  OutputWhenDisabled=reset  InitialOutput=[0]
  cmd_Diverter : Outport  Port=4  OutputWhenDisabled=reset  InitialOutput=[0]
  wires:
  output_pair_open_loop/1 -> hs_Diverter/1
  output_pair_open_loop/2 -> ls_Diverter/1
  output_pair_open_loop/3 -> [state_Diverter], state_Diverter/1
  Diverter/1(cmd_Diverter) -> output_pair_open_loop/1, cmd_Diverter/1
  state_Engine/1 -> output_pair_open_loop/3
  state_Console/1 -> Compare To Constant/1
  Compare To Constant/1 -> output_pair_open_loop/2
  afbk_Diverter/1 -> output_pair_open_loop/4
  ifbk_Diverter/1 -> output_pair_open_loop/5
  calc_Vbattery/1 -> output_pair_open_loop/7
  ain_PrecRef/1 -> output_pair_open_loop/8
  state_Vbattery/1 -> output_pair_open_loop/6
  din_SecondAuxSw/1 -> Diverter/1(din_SecondAuxSw)
  [state_Diverter] -> Unit Delay/1
  Unit Delay/1 -> Diverter/2(state_Diverter)
  mode_LeftControl/1 -> Diverter/3(mode_LeftControl)

== system MX_Gateway/MX_Gtwy_Control/Outputs/Dump_Valve/Dump_Valve ==
  aux_Flow : Inport
  state_Engine : Inport  Port=2
  state_Vbattery : Inport  Port=3
  afbk_DumpValve : Inport  Port=4
  ifbk_DumpValve : Inport  Port=5
  calc_Vbattery : Inport  Port=6
  ain_PrecRef : Inport  Port=7
  Enable : EnablePort  StatesWhenEnabling=reset  PropagateVarSize=During execution
  Compare To Constant : simulink/Logic and Bit Operations/Compare To Constant  relop=~=  const=2  OutDataTypeStr=boolean
  Dump_Valve : Stateflow chart  TreatAsAtomicUnit=on
  hc_pair_control : MX_Library/output_pair_ClosedLoop  AFBK_GAIN=CD_DUMP_VALVE_AFBK_GAIN  IFBK_GAIN=CD_DUMP_VALVE_IFBK_GAIN  OPEN_DB_CNT=CD_DUMP_VALVE_DEBOUNCE_OPEN_CIRCUIT  STG_DB_CNT=CD_DUMP_VALVE_DEBOUNCE_SHORT_TO_GROUND  OSTG_DB_CNT=CD_DUMP_VALVE_DEBOUNCE_SHORT_TO_GROUND  STB_DB_CNT=CD_DUMP_VALVE_DEBOUNCE_SHORT_TO_BATTERY  OC_DB_CNT=CD_DUMP_VALVE_DEBOUNCE_OVERCURRENT  IFBK_MAX=CD_DUMP_VALVE_OVERCURRENT  NZ_IFBK=CD_DUMP_VALVE_NON_ZERO_CURRENT  NZ_DUTY=CD_DUMP_VALVE_NON_ZERO_DUTY  KP=CD_DUMP_VALVE_KP  KI=CD_DUMP_VALVE_KI  BIDIRECTIONAL_CTRL=NON_FUNCTIONAL
  state_DumpValve : Outport  OutputWhenDisabled=reset  InitialOutput=[0]
  hs_DumpValve : Outport  Port=2  OutputWhenDisabled=reset  InitialOutput=[0]
  ls_DumpValve : Outport  Port=3  OutputWhenDisabled=reset  InitialOutput=[0]
  errorCC_DumpValve : Outport  Port=4  OutputWhenDisabled=reset  InitialOutput=[0]
  calc_ifbk_DumpValve : Outport  Port=5  OutputWhenDisabled=reset  InitialOutput=[0]
  wires:
  aux_Flow/1 -> Dump_Valve/1(aux_Flow), Compare To Constant/1
  hc_pair_control/1 -> hs_DumpValve/1
  Dump_Valve/1(icmd_DumpValve) -> hc_pair_control/1
  Compare To Constant/1 -> hc_pair_control/2
  afbk_DumpValve/1 -> hc_pair_control/3
  ifbk_DumpValve/1 -> hc_pair_control/4
  calc_Vbattery/1 -> hc_pair_control/7
  ain_PrecRef/1 -> hc_pair_control/8
  state_Vbattery/1 -> hc_pair_control/5
  hc_pair_control/2 -> ls_DumpValve/1
  hc_pair_control/5 -> state_DumpValve/1
  state_Engine/1 -> hc_pair_control/6
  hc_pair_control/3 -> errorCC_DumpValve/1
  hc_pair_control/4 -> calc_ifbk_DumpValve/1

== system MX_Gateway/MX_Gtwy_Control/Outputs/FuelPri_HVAC/Fuel_Primary_Relay ==
  dfbk_FuelPriOutput : Inport  OutDataTypeStr=boolean
  din_FuelPriRelay : Inport  Port=2  OutDataTypeStr=boolean
  error_FuelSec : Inport  Port=3  OutDataTypeStr=uint8
  state_Engine : Inport  Port=4
  flt_ECT : Inport  Port=5  OutDataTypeStr=uint16
  timer_Resolution : Inport  Port=6  OutDataTypeStr=uint8
  state_VBattery : Inport  Port=7
  Compare To Constant : simulink/Logic and Bit Operations/Compare To Constant  relop=~=  const=NON_FUNCTIONAL  OutDataTypeStr=boolean  ZeroCross=off
  Compare To Constant1 : simulink/Logic and Bit Operations/Compare To Constant  relop=~=  const=OORL  OutDataTypeStr=boolean  ZeroCross=off
  FunctionState : Constant  Value=CD_FUEL_PRIMARY_FEEDBACK_FUNCTION  OutDataTypeStr=uint8
  Logical Operator : Logic  AllPortsSameDT=off  OutDataTypeStr=boolean
  PullTime1 : Lookup  InputValues=0:8:336  Table=MXGTW_FUEL_PULL_TIME  LookUpMeth=Use Input Nearest  OutDataTypeStr=uint16  RndMeth=Nearest
      settings: AttributesFormatString=input = %<InputValues>\noutput = %<OutputValues>\nlookup = %<LookUpMeth>\nrounding = %<RndMeth>
  RelaySolenoid : Stateflow chart  TreatAsAtomicUnit=on
  Unit Delay1 : UnitDelay  SampleTime=-1
      settings: AttributesFormatString=<initial=%<X0>>\n<tsample=%<SampleTime>>
  Unit Delay2 : UnitDelay  SampleTime=-1
      settings: AttributesFormatString=<initial=%<X0>>\n<tsample=%<SampleTime>>
  relay_oc : MX_Library/relay_oc  ERROR_ON_DB_CNT=CD_FUEL_PRIMARY_DEBOUNCE_ERROR_ON  ERROR_OFF_DB_CNT=CD_FUEL_PRIMARY_DEBOUNCE_ERROR_OFF  NORMAL_DB_CNT=CD_FUEL_PRIMARY_DEBOUNCE_NORMAL
  dout_FuelPri : Outport  OutDataTypeStr=boolean
  state_FuelPriOutput : Outport  Port=2  OutDataTypeStr=uint8
  state_FuelPriRelay : Outport  Port=3  OutDataTypeStr=uint8
  wires:
  timer_Resolution/1 -> RelaySolenoid/3(timer_Resolution)  "timer_Resolution"
  flt_ECT/1 -> PullTime1/1  "flt_ECT"
  state_Engine/1 -> RelaySolenoid/5(state_Engine)  "state_Engine"
  Logical Operator/1 -> relay_oc/Enable  "EnableFeedback"
  Compare To Constant1/1 -> Logical Operator/2
  Compare To Constant/1 -> Logical Operator/1
  state_VBattery/1 -> Compare To Constant1/1  "state_VBattery"
  FunctionState/1 -> Compare To Constant/1
  relay_oc/1 -> Unit Delay1/1, state_FuelPriOutput/1  "state_FuelPriOutput"
  Unit Delay2/1 -> RelaySolenoid/2(state_RelayError)  "state_RelayError"
  Unit Delay1/1 -> RelaySolenoid/1(state_OutputError)  "state_OutputError"
  relay_oc/2 -> Unit Delay2/1, state_FuelPriRelay/1  "state_FuelPriRelay"
  din_FuelPriRelay/1 -> relay_oc/3  "din_FuelPriRelay"
  dfbk_FuelPriOutput/1 -> relay_oc/2  "dfbk_FuelPriOutput"
  RelaySolenoid/1(dout_FuelPri) -> dout_FuelPri/1, relay_oc/1  "dout_FuelPri"
  error_FuelSec/1 -> RelaySolenoid/4(error_FuelSec)  "error_FuelSec"
  PullTime1/1 -> RelaySolenoid/6(PullTime)  "time_Pull"

== system MX_Gateway/MX_Gtwy_Control/Outputs/FuelPri_HVAC/HVAC_Relay ==
  state_Engine : Inport  OutDataTypeStr=uint8
  dfbk_HVACOutput : Inport  Port=2
  state_Vbattery : Inport  Port=3
  state_System : Inport  Port=4  OutDataTypeStr=uint8
  Compare To Constant2 : simulink/Logic and Bit Operations/Compare To Constant  relop=~=  const=NON_FUNCTIONAL  OutDataTypeStr=boolean  ZeroCross=off
  Compare To Constant3 : simulink/Logic and Bit Operations/Compare To Constant  relop=~=  const=OORL  OutDataTypeStr=boolean  ZeroCross=off
  FunctionState1 : Constant  Value=CD_HVAC_RELAY_FEEDBACK_FUNCTION  OutDataTypeStr=uint8
  Logical Operator1 : Logic  AllPortsSameDT=off  OutDataTypeStr=boolean
  RelaySolenoid : Stateflow chart  TreatAsAtomicUnit=on
  Unit Delay3 : UnitDelay  SampleTime=-1
      settings: AttributesFormatString=<initial=%<X0>>\n<tsample=%<SampleTime>>
  dout_chk : MX_Library/dout_chk  ERR_ON_DB_CNT=CD_HVAC_RELAY_DEBOUNCE_ERROR_ON  ERR_OFF_DB_CNT=CD_HVAC_RELAY_DEBOUNCE_ERROR_OFF
  dout_HVACOutput : Outport  OutDataTypeStr=boolean
  state_HVACOutput : Outport  Port=2  OutDataTypeStr=uint8
  wires:
  RelaySolenoid/1(dout_HVACRelay) -> dout_HVACOutput/1, dout_chk/1
  dfbk_HVACOutput/1 -> dout_chk/2
  state_Engine/1 -> RelaySolenoid/2(state_Engine)  "state_Engine"
  state_System/1 -> RelaySolenoid/3(state_System)  "state_System"
  state_Vbattery/1 -> [state_Vbattery]
  dout_chk/1 -> [state_Output], state_HVACOutput/1
  Compare To Constant3/1 -> Logical Operator1/2  "EnableFeedback"
  Logical Operator1/1 -> dout_chk/Enable
  FunctionState1/1 -> Compare To Constant2/1
  Compare To Constant2/1 -> Logical Operator1/1
  Unit Delay3/1 -> RelaySolenoid/1(state_OutputError)
  [state_Output] -> Unit Delay3/1
  [state_Vbattery] -> Compare To Constant3/1

== system MX_Gateway/MX_Gtwy_Control/Outputs/Hyd_Bypass/Hyd_Bypass ==
  state_Engine : Inport
  state_Console : Inport  Port=2
  state_Vbattery : Inport  Port=3
  afbk_HydBypass : Inport  Port=4
  ifbk_HydBypass : Inport  Port=5
  calc_Vbattery : Inport  Port=6
  ain_PrecRef : Inport  Port=7
  Enable : EnablePort  StatesWhenEnabling=reset  PropagateVarSize=During execution
  Compare To Constant : simulink/Logic and Bit Operations/Compare To Constant  relop=~=  const=ACTIVE  OutDataTypeStr=boolean
  Hyd_Bypass : Stateflow chart  TreatAsAtomicUnit=on
  output_pair_open_loop : MX_Library/output_pair_OpenLoop  FEEDBACK_ENABLE=CD_HYD_BYPASS_FEEDBACK_FUNCTION  AFBK_GAIN=CD_HYD_BYPASS_AFBK_GAIN  IFBK_GAIN=CD_HYD_BYPASS_IFBK_GAIN  OPEN_DB_CNT=CD_HYD_BYPASS_DEBOUNCE_OPEN_CIRCUIT  STG_DB_CNT=CD_HYD_BYPASS_DEBOUNCE_SHORT_TO_GROUND  STB_DB_CNT=CD_HYD_BYPASS_DEBOUNCE_SHORT_TO_BATTERY  OC_DB_CNT=CD_HYD_BYPASS_DEBOUNCE_OVERCURRENT  OSTG_DB_CNT=CD_HYD_BYPASS_DEBOUNCE_SHORT_TO_GROUND  IFBK_MAX=CD_HYD_BYPASS_OVERCURRENT  NZ_IFBK=CD_HYD_BYPASS_NON_ZERO_CURRENT  NZ_DUTY=CD_HYD_BYPASS_NON_ZERO_DUTY  MAX_VOLTS=CD_HYD_BYPASS_MAX_VOLTS
  state_HydBypass : Outport  OutputWhenDisabled=reset  InitialOutput=[0]
  hs_HydBypass : Outport  Port=2  OutputWhenDisabled=reset  InitialOutput=[0]
  ls_HydBypass : Outport  Port=3  OutputWhenDisabled=reset  InitialOutput=[0]
  wires:
  state_Engine/1 -> Hyd_Bypass/1(state_Engine), [state_Engine]
  state_Console/1 -> Hyd_Bypass/2(state_Console), [state_Console]
  output_pair_open_loop/1 -> hs_HydBypass/1
  output_pair_open_loop/2 -> ls_HydBypass/1
  output_pair_open_loop/3 -> state_HydBypass/1
  Hyd_Bypass/1(dout_HydBypass) -> output_pair_open_loop/1
  [state_Engine] -> output_pair_open_loop/3
  [state_Console] -> Compare To Constant/1
  Compare To Constant/1 -> output_pair_open_loop/2
  afbk_HydBypass/1 -> output_pair_open_loop/4
  ifbk_HydBypass/1 -> output_pair_open_loop/5
  calc_Vbattery/1 -> output_pair_open_loop/7
  ain_PrecRef/1 -> output_pair_open_loop/8
  state_Vbattery/1 -> output_pair_open_loop/6

== system MX_Gateway/MX_Gtwy_Control/Outputs/Hyd_Enable/Hyd_Enable ==
  state_Engine : Inport
  state_Console : Inport  Port=2
  state_Vbattery : Inport  Port=3
  afbk_HydEnable : Inport  Port=4
  ifbk_HydEnable : Inport  Port=5
  calc_Vbattery : Inport  Port=6
  ain_PrecRef : Inport  Port=7
  state_Inverter : Inport  Port=8
  Enable : EnablePort  StatesWhenEnabling=reset  PropagateVarSize=During execution
  Compare To Constant : simulink/Logic and Bit Operations/Compare To Constant  relop=~=  const=ACTIVE  OutDataTypeStr=boolean
  Constant : Constant  Value=CD_EMEA_ELECTRIC_VERSION  OutDataTypeStr=uint8
  Hyd_Enable : Stateflow chart  TreatAsAtomicUnit=on
  Switch : Switch
  output_pair_open_loop : MX_Library/output_pair_OpenLoop  FEEDBACK_ENABLE=CD_HYD_ENABLE_FEEDBACK_FUNCTION  AFBK_GAIN=CD_HYD_ENABLE_AFBK_GAIN  IFBK_GAIN=CD_HYD_ENABLE_IFBK_GAIN  OPEN_DB_CNT=CD_HYD_ENABLE_DEBOUNCE_OPEN_CIRCUIT  STG_DB_CNT=CD_HYD_ENABLE_DEBOUNCE_SHORT_TO_GROUND  STB_DB_CNT=CD_HYD_ENABLE_DEBOUNCE_SHORT_TO_BATTERY  OC_DB_CNT=CD_HYD_ENABLE_DEBOUNCE_OVERCURRENT  OSTG_DB_CNT=CD_HYD_ENABLE_DEBOUNCE_SHORT_TO_GROUND  IFBK_MAX=CD_HYD_ENABLE_OVERCURRENT  NZ_IFBK=CD_HYD_ENABLE_NON_ZERO_CURRENT  NZ_DUTY=CD_HYD_ENABLE_NON_ZERO_DUTY  MAX_VOLTS=CD_HYD_ENABLE_MAX_VOLTS
  state_HydEnable : Outport  OutputWhenDisabled=reset  InitialOutput=[0]
  hs_HydEnable : Outport  Port=2  OutputWhenDisabled=reset  InitialOutput=[0]
  ls_HydEnable : Outport  Port=3  OutputWhenDisabled=reset  InitialOutput=[0]
  wires:
  state_Engine/1 -> Hyd_Enable/1(state_Engine), [state_Engine]
  state_Console/1 -> Hyd_Enable/2(state_Console), [state_Console]
  output_pair_open_loop/1 -> hs_HydEnable/1
  output_pair_open_loop/2 -> ls_HydEnable/1
  output_pair_open_loop/3 -> state_HydEnable/1
  Hyd_Enable/1(dout_HydEnable) -> Switch/3
  [state_Engine] -> output_pair_open_loop/3
  [state_Console] -> Compare To Constant/1
  Compare To Constant/1 -> output_pair_open_loop/2
  afbk_HydEnable/1 -> output_pair_open_loop/4
  ifbk_HydEnable/1 -> output_pair_open_loop/5
  calc_Vbattery/1 -> output_pair_open_loop/7
  ain_PrecRef/1 -> output_pair_open_loop/8
  state_Vbattery/1 -> output_pair_open_loop/6
  state_Inverter/1 -> Switch/1
  Switch/1 -> output_pair_open_loop/1
  Constant/1 -> Switch/2
